simulink model "ofdm_txrx_supermimo_coded"  (Simulink 7.1)
settings: Solver=VariableStepDiscrete  SolverMode=SingleTasking  StartTime=0.0  StopTime=2*simOnly_simLength  RelTol=1e-3
PostLoadFcn:
  | ofdm_rx_supermimo_init
InitFcn:
  | ofdm_rx_supermimo_init
StartFcn:
  | ofdm_rx_supermimo_init
values from neighbouring files (symbols this model uses):
  Action0_Config = 0* 31*2^18 + 0*2^24 + PHY_AUTORESPONSE_REQ_GOODHDR + PHY_AUTORESPONSE_REQ_GOODPKT + PHY_AUTORESPONSE_REQ_FLAGA    [ofdm_tx_supermimo_init.m]
  Action1_Config = 0* 62*2^18 + PHY_AUTORESPONSE_REQ_GOODHDR + PHY_AUTORESPONSE_REQ_GOODPKT    [ofdm_tx_supermimo_init.m]
  Action2_Config = 0    [ofdm_tx_supermimo_init.m]
  Action3_Config = 0    [ofdm_tx_supermimo_init.m]
  Action4_Config = 0    [ofdm_tx_supermimo_init.m]
  Action5_Config = 0    [ofdm_tx_supermimo_init.m]
  ActionID_AFTransmit = 31    [ofdm_tx_supermimo_init.m]
  ActionID_Disabled = 0    [ofdm_tx_supermimo_init.m]
  ActionID_SetFlagA = 62    [ofdm_tx_supermimo_init.m]
  ActionID_SetFlagB = 61    [ofdm_tx_supermimo_init.m]
  BER_RAM_init_size = 2048    [ofdm_tx_supermimo_init.m]
  channel_h = [1 0; 1 0] * 0.3    [ofdm_tx_supermimo_init.m]
  CPLength = 16    [ofdm_tx_supermimo_init.m]
  FEC_Config = fec_coding_en*1 + fec_soft_dec*2 + fec_qpsk_scl*2^4 + fec_16qam_scl*2^8    (= 4195)    [ofdm_tx_supermimo_init.m]
  longCorr_coef_i = [-4*real(LTS) 0]    [ofdm_rx_supermimo_init.m]
  longCorr_coef_q = [4*imag(LTS) 0]    [ofdm_rx_supermimo_init.m]
  Match0_Config = 0    [ofdm_tx_supermimo_init.m]
  Match1_Config = 0    [ofdm_tx_supermimo_init.m]
  Match2_Config = 0    [ofdm_tx_supermimo_init.m]
  Match3_Config = 0    [ofdm_tx_supermimo_init.m]
  Match4_Config = 0    [ofdm_tx_supermimo_init.m]
  Match5_Config = 0    [ofdm_tx_supermimo_init.m]
  Match6_Config = 0    [ofdm_tx_supermimo_init.m]
  Match7_Config = 0    [ofdm_tx_supermimo_init.m]
  max_CP_length = 16    [ofdm_tx_supermimo_init.m]
  max_num_baseRateSymbols = 31    [ofdm_tx_supermimo_init.m]
  max_num_subcarriers = 64    [ofdm_tx_supermimo_init.m]
  max_num_trainingSymbols = 15    [ofdm_tx_supermimo_init.m]
  max_numBytes = 16384    [ofdm_tx_supermimo_init.m]
  max_OFDM_symbols = 1023    [ofdm_tx_supermimo_init.m]
  modConstellation_qam16 = .75*[1 3 -1 -3]./3    [ofdm_tx_supermimo_init.m]
  modConstellation_qam64 = 0.875*[3 1 5 7 -3 -1 -5 -7]./7    [ofdm_tx_supermimo_init.m]
  modConstellation_qpsk = [1 -1]./sqrt(2)    [ofdm_tx_supermimo_init.m]
  numBaseRateSymbols = numBaseRateSymbols *2    [ofdm_tx_supermimo_init.m]
  numSubcarriers = 64    [ofdm_tx_supermimo_init.m]
  numTrainingSymbols = 2    [ofdm_tx_supermimo_init.m]
  pilot_indicies = 7 + ( (64-21) * 2^8) + ( (64-7) * 2^16) + (21 * 2^24)    (= 356068103)    [ofdm_tx_supermimo_init.m]
  pilotValues = hex2dec('A57D') + (2^16 * hex2dec('5A82'))    [ofdm_tx_supermimo_init.m]
  pktByteNums = numHeaderBytes +  				byteIndex_numPayloadBytes(1)*2^8 +  				byteIndex_numPayloadBytes(2)*2^16 +  				byteIndex_simpleDynModMasks * 2^24    [ofdm_rx_supermimo_init.m]
  PktDet_Delay = 58+32 +      Symbol_Timing_Offset*2^7 +      0*2^16 +      (117* 2^24)    (= 1962935642)    [ofdm_rx_supermimo_init.m]
  pktTiming_count_payloadStart = pktTiming_count_lsStart + pktTiming_ls_num*pktTiming_ls_length    [ofdm_rx_supermimo_init.m]
  preamble = preamble_scale*[shortsyms_10 longsyms_2]    [ofdm_tx_supermimo_init.m]
  preamble_ROM = preamble_I_ROM + preamble_Q_ROM*2^16    [ofdm_tx_supermimo_init.m]
  RAM_init_size = max_numBytes    (= 16384)    [ofdm_tx_supermimo_init.m]
  reg_AF_Tx_Blanking = (320+80) + ( (320+80+79)*2^16)    (= 31392144)    [ofdm_rx_supermimo_init.m]
  reg_AF_Tx_Scaling = 2944    [ofdm_rx_supermimo_init.m]
  reg_Interrupt_PktBuf_Control = reg_InterruptControl +  	1 * 2^16 +  	0 * 2^24    (= 65536)    [ofdm_rx_supermimo_init.m]
  reg_PilotCalcParams = (4 * 2^16) + (minPilotChanMag)    (= 262184.96)    [ofdm_rx_supermimo_init.m]
  regRx_chanEst_minMag = ((0.5)*2^15) + ((0.5 * 2^15)*2^16)    (= 1073758208)    [ofdm_rx_supermimo_init.m]
  regRx_coarseCalcCorrection = round((1.3e-3 - 1.2982e-3)*2^32)    [ofdm_rx_supermimo_init.m]
  regRx_pilotCalcCorrection = round(1.00115 * 2^31)    [ofdm_rx_supermimo_init.m]
  regRx_preCFOoptions = 0 * 2^0 +  	1 * 2^1 +  	0    (= 2)    [ofdm_rx_supermimo_init.m]
  rx_controlBits = 1 * 2  		 + 1 * 4  		 + 0 * 8  		 + 1 * 16 * rx_SISO_Mode  		 + 1 * 32  		 + 1 * 64  		 + 1 * 128  		 + 1 * 256  		 + 1 * 512  		 + 1 * 1024  		 + 1 * 4096  		 + 0 * 16384  		 + 0 * 32768  		 + 1 * 65536  		 + 0 * 131072  		 + 0 * 262144  		 + 1 * 524288  		 + 1 * 1048576 * tx_Alamouti_Mode  		 + 0 * 2097152           + 1 * 33554432  		 + 0 * 4194304  		 + 0 * 8388608  		 + 0 * 16777216  		 + 1 *  ...(+49 chars)    [ofdm_rx_supermimo_init.m]
  Rx_PktDet_LongCorr_Params = LongCorrCounterLoad + 0*2^8 + 0*2^16 + 255*2^24    (= 4278190325)    [ofdm_rx_supermimo_init.m]
  Rx_PktDet_LongCorrThresholds = 8000    [ofdm_rx_supermimo_init.m]
  rx_postEq_scaling = round(rxScaling*2^11) * (1 + 2^16)    [ofdm_rx_supermimo_init.m]
  RxDataScrambling_Seq = [40 198 78 63 82 173 102 245 48 111 172 115 147 230 216 93 72 65 62 2 205 242 122 90 128 83 105 97 73 10 5 252]    [ofdm_tx_supermimo_init.m]
  RxReg_FixedPktLen = 0    [ofdm_rx_supermimo_init.m]
  rxReg_pktDetCorr = 2^00 * (floor(0.7*128)) +  	2^08 * (300) +  	0    [ofdm_rx_supermimo_init.m]
  shortSymbol_time = shortSymbol_time(1:16).'    [ofdm_tx_supermimo_init.m]
  simOnly_numSamples = length(preamble)+( (numSubcarriers+CPLength)*(numTrainingSymbols + numBaseRateSymbols + numFullRateSymbols/2) )    [ofdm_tx_supermimo_init.m]
  train = [train train]    [ofdm_tx_supermimo_init.m]
  tx_controlBits = (antB_preambleShift * 2^4) + (2^1 * tx_Alamouti_Mode) +  	(2^0 * tx_SISO_Mode) + 4*0 + 8*1 + 0*256 + 512*0 + 1024*0 +  	0*hex2dec('1000') + 0*hex2dec('2000') + 0*hex2dec('4000') +  	1*hex2dec('8000') + 1*hex2dec('10000') + hex2dec('20000') +  	0*hex2dec('100000')    [ofdm_tx_supermimo_init.m]
  tx_delays = (50*2^0) + (0*2^8) + (30*2^12)    (= 122930)    [ofdm_tx_supermimo_init.m]
  tx_scaling = (3072) + (12288 * 2^16)    (= 805309440)    [ofdm_tx_supermimo_init.m]
  TxDataScrambling_Seq = [40 198 78 63 82 173 102 245 48 111 172 115 147 230 216 93 72 65 62 2 205 242 122 90 128 83 105 97 73 10 5 252]    [ofdm_tx_supermimo_init.m]
  txReg_symbolCounts = (2^8 *numBaseRateSymbols ) + numTrainingSymbols    [ofdm_tx_supermimo_init.m]
  TxRx_FFTScaling = (3 * 2^0) +  	(2 * 2^2) +  	(1 * 2^4) +  	(1 * 2^6) +  	(1 * 2^8) +  	(0 * 2^10)    (= 347)    [ofdm_tx_supermimo_init.m]
undefined symbols (not defined in the file or in its folder): AntA, AntB, BaseRate, Down, gcbh, numBuffs, numBytes, Up, xlBlockLoadCallback, xlGetSimulinkPeriod, xlipgetpartsetting
libraries: xbsIndex_r4 x6000, simulink x4, dspsigops x4, commchan3 x2, dspsrcs4 x2, commrflib2 x2
(part 40 of 63)

== system ofdm_txrx_supermimo_coded/OFDM Tx MIMO/Training_Data/FlexibleMod/PktBuffer_CRC1/CRC32 Calc ==
  ByteIn : Inport  OutDataType=sfix(16)  OutScaling=2^0
  Vin : Inport  Orientation=left  Port=2  OutDataType=sfix(16)  OutScaling=2^0
  Rst : Inport  Orientation=left  Port=3  OutDataType=sfix(16)  OutScaling=2^0
  Capt : Inport  Port=4  OutDataType=sfix(16)  OutScaling=2^0
  24LSB : xbsIndex_r4/Slice  nbits=24  boolean_output=off  mode=Lower Bit Location + Width  bit1=0  base1=MSB of Input  bit0=0  base0=LSB of Input  dbl_ovrd=off  block_type=slice
      settings: infoedit="Extracts a given range of bits from each input sample and presents it at the output.  The output type is ordinarily unsigned with binary point at zero, but can be Boolean when the slice is one bit wide.<P><P>Hardware notes: In hardware this block costs nothing."  block_version=VER_STRING_GOES_HERE
  8MSB : xbsIndex_r4/Slice  nbits=8  boolean_output=off  mode=Upper Bit Location + Width  bit1=0  base1=MSB of Input  bit0=0  base0=LSB of Input  dbl_ovrd=off  block_type=slice
      settings: infoedit="Extracts a given range of bits from each input sample and presents it at the output.  The output type is ordinarily unsigned with binary point at zero, but can be Boolean when the slice is one bit wide.<P><P>Hardware notes: In hardware this block costs nothing."  block_version=VER_STRING_GOES_HERE
  Assert : xbsIndex_r4/Assert  Orientation=left  assert_type=off  type_source=Explicitly  arith_type="Signed  (2's comp)"  n_bits=16  bin_pt=14  assert_rate=on  rate_source=Explicitly  period=2  output_port=on  block_type=assert
      settings: infoedit=Asserts a user-defined sample rate and/or type on a signal.<P><P>Hardware notes: In hardware this block costs nothing.  block_version=VER_STRING_GOES_HERE
  CRC Remainders : xbsIndex_r4/ROM  depth=256  initVector=CRC_Table32  distributed_mem=Distributed memory  rst=off  init_reg=0  en=off  latency=0  arith_type=Unsigned  n_bits=32  bin_pt=0  dbl_ovrd=off  optimize=Area  use_rpm=off  block_type=sprom
      settings: xl_area=[0, 0, 0, 0, 0, 0, 0]  block_version=VER_STRING_GOES_HERE
  Concat : xbsIndex_r4/Concat  num_inputs=2  dbl_ovrd=off  block_type=concat
      settings: block_version=VER_STRING_GOES_HERE
  Constant : xbsIndex_r4/Constant  arith_type=Unsigned  const=0  n_bits=8  bin_pt=0  explicit_period=off  period=1  equ=P=C  opselect=C  inp2=PCIN>>17  opr=+  inp1=P  carry=CIN  dbl_ovrd=off  block_type=constant
      settings: block_version=VER_STRING_GOES_HERE
  Delay : xbsIndex_r4/Delay  en=off  latency=1  dbl_ovrd=off  reg_retiming=off  block_type=delay
      settings: infoedit=Hardware notes: A delay line is a chain, each link of which is an SRL16 followed by a flip-flop. If register retiming is enabled, the delay line is a chain of flip-flops.  block_version=8.2.01
  Logical1 : xbsIndex_r4/Logical  logical_function=XOR  inputs=2  en=off  latency=0  precision=Full  arith_type=Unsigned  n_bits=8  bin_pt=2  align_bp=on  dbl_ovrd=off  block_type=logical
      settings: xl_area=[0, 0, 0, 0, 0, 0, 0]  block_version=VER_STRING_GOES_HERE
  Logical2 : xbsIndex_r4/Logical  Orientation=left  logical_function=XOR  inputs=2  en=off  latency=0  precision=Full  arith_type=Unsigned  n_bits=8  bin_pt=2  align_bp=on  dbl_ovrd=off  block_type=logical
      settings: xl_area=[0, 0, 0, 0, 0, 0, 0]  block_version=VER_STRING_GOES_HERE
  Logical4 : xbsIndex_r4/Logical  logical_function=AND  inputs=2  en=off  latency=0  precision=Full  arith_type=Unsigned  n_bits=8  bin_pt=2  align_bp=on  dbl_ovrd=off  block_type=logical
      settings: xl_area=[1 0 0 1 0 0 0]  block_version=VER_STRING_GOES_HERE
  crc_accum : xbsIndex_r4/Register  Orientation=left  init=hex2dec('FFFFFFFF')  rst=on  en=on  dbl_ovrd=off  block_type=register
      settings: xl_area=[0, 0, 0, 0, 0, 0, 0]  block_version=VER_STRING_GOES_HERE
  crc_capt : xbsIndex_r4/Register  init=0  rst=on  en=on  dbl_ovrd=off  block_type=register
      settings: xl_area=[0, 0, 0, 0, 0, 0, 0]  block_version=VER_STRING_GOES_HERE
  32-bit CRC Capt : Outport  OutDataType=sfix(16)  OutScaling=2^0
  wires:
  8MSB/1 -> Logical1/1
  crc_accum/1 -> Assert/1
  Logical1/1 -> CRC Remainders/1
  Logical2/1 -> crc_accum/1, crc_capt/1
  24LSB/1 -> Concat/1
  Constant/1 -> Concat/2
  Concat/1 -> Logical2/1
  CRC Remainders/1 -> Logical2/2
  Rst/1 -> crc_accum/2, crc_capt/2
  ByteIn/1 -> Logical1/2
  Vin/1 -> crc_accum/3, Logical4/2
  Assert/1 -> 24LSB/1, 8MSB/1
  Logical4/1 -> Delay/1
  Capt/1 -> Logical4/1
  crc_capt/1 -> 32-bit CRC Capt/1
  Delay/1 -> crc_capt/3

== system ofdm_txrx_supermimo_coded/OFDM Tx MIMO/Training_Data/FlexibleMod/PktBuffer_CRC1/Header Decoding1 ==
  TxByteAddr : Inport  OutDataType=sfix(16)  OutScaling=2^0
  TxByte : Inport  Port=2  OutDataType=sfix(16)  OutScaling=2^0
  TxByteAddrVin : Inport  Port=3  OutDataType=sfix(16)  OutScaling=2^0
  Rst : Inport  Port=4  OutDataType=sfix(16)  OutScaling=2^0
  4LSB : xbsIndex_r4/Slice  nbits=4  boolean_output=off  mode=Lower Bit Location + Width  bit1=0  base1=MSB of Input  bit0=0  base0=LSB of Input  dbl_ovrd=off  block_type=slice
      settings: infoedit="Extracts a given range of bits from each input sample and presents it at the output.  The output type is ordinarily unsigned with binary point at zero, but can be Boolean when the slice is one bit wide.<P><P>Hardware notes: In hardware this block costs nothing."  block_version=VER_STRING_GOES_HERE
  4MSB : xbsIndex_r4/Slice  nbits=4  boolean_output=off  mode=Upper Bit Location + Width  bit1=0  base1=MSB of Input  bit0=0  base0=LSB of Input  dbl_ovrd=off  block_type=slice
      settings: infoedit="Extracts a given range of bits from each input sample and presents it at the output.  The output type is ordinarily unsigned with binary point at zero, but can be Boolean when the slice is one bit wide.<P><P>Hardware notes: In hardware this block costs nothing."  block_version=VER_STRING_GOES_HERE
  8LSB : xbsIndex_r4/Slice  nbits=8  boolean_output=off  mode=Lower Bit Location + Width  bit1=0  base1=MSB of Input  bit0=0  base0=LSB of Input  dbl_ovrd=off  block_type=slice
      settings: infoedit="Extracts a given range of bits from each input sample and presents it at the output.  The output type is ordinarily unsigned with binary point at zero, but can be Boolean when the slice is one bit wide.<P><P>Hardware notes: In hardware this block costs nothing."  block_version=VER_STRING_GOES_HERE
  8LSB+8 : xbsIndex_r4/Slice  nbits=8  boolean_output=off  mode=Lower Bit Location + Width  bit1=0  base1=MSB of Input  bit0=8  base0=LSB of Input  dbl_ovrd=off  block_type=slice
      settings: infoedit="Extracts a given range of bits from each input sample and presents it at the output.  The output type is ordinarily unsigned with binary point at zero, but can be Boolean when the slice is one bit wide.<P><P>Hardware notes: In hardware this block costs nothing."  block_version=VER_STRING_GOES_HERE
  8MSB : xbsIndex_r4/Slice  nbits=8  boolean_output=off  mode=Upper Bit Location + Width  bit1=0  base1=MSB of Input  bit0=0  base0=LSB of Input  dbl_ovrd=off  block_type=slice
      settings: infoedit="Extracts a given range of bits from each input sample and presents it at the output.  The output type is ordinarily unsigned with binary point at zero, but can be Boolean when the slice is one bit wide.<P><P>Hardware notes: In hardware this block costs nothing."  block_version=VER_STRING_GOES_HERE
  Assert1 : xbsIndex_r4/Assert  assert_type=off  type_source=Explicitly  arith_type="Signed  (2's comp)"  n_bits=16  bin_pt=14  assert_rate=on  rate_source=Explicitly  period=2  output_port=on  block_type=assert
      settings: infoedit=Asserts a user-defined sample rate and/or type on a signal.<P><P>Hardware notes: In hardware this block costs nothing.  block_version=VER_STRING_GOES_HERE
  Assert2 : xbsIndex_r4/Assert  assert_type=off  type_source=Explicitly  arith_type="Signed  (2's comp)"  n_bits=16  bin_pt=14  assert_rate=on  rate_source=Explicitly  period=2  output_port=on  block_type=assert
      settings: infoedit=Asserts a user-defined sample rate and/or type on a signal.<P><P>Hardware notes: In hardware this block costs nothing.  block_version=VER_STRING_GOES_HERE
  Logical1 : xbsIndex_r4/Logical  logical_function=AND  inputs=2  en=off  latency=0  precision=Full  arith_type=Unsigned  n_bits=8  bin_pt=2  align_bp=on  dbl_ovrd=off  block_type=logical
      settings: xl_area=[1 0 0 1 0 0 0]  block_version=VER_STRING_GOES_HERE
  Logical2 : xbsIndex_r4/Logical  logical_function=AND  inputs=2  en=off  latency=0  precision=Full  arith_type=Unsigned  n_bits=8  bin_pt=2  align_bp=on  dbl_ovrd=off  block_type=logical
      settings: xl_area=[1 0 0 1 0 0 0]  block_version=VER_STRING_GOES_HERE
  Logical4 : xbsIndex_r4/Logical  logical_function=AND  inputs=2  en=off  latency=0  precision=Full  arith_type=Unsigned  n_bits=8  bin_pt=2  align_bp=on  dbl_ovrd=off  block_type=logical
      settings: xl_area=[1 0 0 1 0 0 0]  block_version=VER_STRING_GOES_HERE
  Register1 : xbsIndex_r4/Register  init=34  rst=on  en=on  dbl_ovrd=off  block_type=register
      settings: xl_area=[5 10 0 0 0 0 0]  block_version=VER_STRING_GOES_HERE
  Register2 : xbsIndex_r4/Register  init=0  rst=on  en=off  dbl_ovrd=off  block_type=register
      settings: xl_area=[5 10 0 0 0 0 0]  block_version=VER_STRING_GOES_HERE
  Relational1 : xbsIndex_r4/Relational  mode=a=b  en=off  latency=0  dbl_ovrd=off  block_type=relational
      settings: xl_area=[6 0 0 11 0 0 0]  block_version=VER_STRING_GOES_HERE
  Relational2 : xbsIndex_r4/Relational  mode=a=b  en=off  latency=0  dbl_ovrd=off  block_type=relational
      settings: xl_area=[6 0 0 11 0 0 0]  block_version=VER_STRING_GOES_HERE
  Relational3 : xbsIndex_r4/Relational  mode=a=b  en=off  latency=0  dbl_ovrd=off  block_type=relational
      settings: xl_area=[6 0 0 11 0 0 0]  block_version=VER_STRING_GOES_HERE
  numBytesUpdate : Subsystem
  numBytes : Outport  OutDataType=sfix(16)  OutScaling=2^0
  modMask : Outport  Port=2  OutDataType=sfix(16)  OutScaling=2^0
  wires:
  Relational3/1 -> Logical2/2
  8LSB+8/1 -> Relational3/2
  Relational2/1 -> Logical4/2
  8LSB/1 -> Relational2/2
  Rst/1 -> Register1/2, numBytesUpdate/1(rst), Register2/2
  Logical4/1 -> numBytesUpdate/3(lsb_EN)
  Logical2/1 -> numBytesUpdate/4(msb_EN)
  numBytesUpdate/1(numBytes) -> Assert1/1
  Assert1/1 -> numBytes/1
  [regRx_headerByteNums] -> 8LSB+8/1, 8LSB/1, 8MSB/1
  TxByteAddrVin/1 -> Logical4/1, Logical1/1, Logical2/1
  TxByte/1 -> numBytesUpdate/2(d), Register1/1
  Register2/1 -> Relational2/1, Relational3/1, Relational1/1
  Relational1/1 -> Logical1/2
  Logical1/1 -> Register1/3
  8MSB/1 -> Relational1/2
  Register1/1 -> Assert2/1
  Assert2/1 -> modMask/1, 4LSB/1, 4MSB/1
  4LSB/1 -> numBytesUpdate/6(modMask_AntB)
  4MSB/1 -> numBytesUpdate/5(modMask_AntA)
  TxByteAddr/1 -> Register2/1

== system ofdm_txrx_supermimo_coded/OFDM Tx MIMO/Training_Data/FlexibleMod/PktBuffer_CRC1/Packet Buffer ==
  note: For real-time BER testing, the Rx block uses this logic
      | to access the packet buffer. In hardware, Tx/Rx is half-duplex,
      | so sharing the logic is no problem. In simulation, it's full-duplex
      | (loopback, really), so some muxing is required.
  note: Use a long (12-bit) LFSR for more random payloads
      | Delay the Mux select by 1 cycle to line up with the latency
      | of the actual packet buffer (don't want to randomize the last
      | byte of the header!)
      | Polynomial set to dec2hex(2^11+2^8+2^6+2^0),
      | the optimal for a 12-bit LFSR per xapp210.
  note: Increment the LFSR on each new byte address. This
      | logic will "miss" byte0, but since we don't record
      | headers for random payloads, it doesn't matter.
  note: Disable random payload generation for
      | automatically transmitted packets
      | (either via autoTwoTx or via
      | autoResponders)
  TxByteAddr : Inport  OutDataType=sfix(16)  OutScaling=2^0
  1LSB : xbsIndex_r4/Slice  nbits=1  boolean_output=on  mode=Lower Bit Location + Width  bit1=0  base1=MSB of Input  bit0=0  base0=LSB of Input  dbl_ovrd=off  block_type=slice
      settings: infoedit="Extracts a given range of bits from each input sample and presents it at the output.  The output type is ordinarily unsigned with binary point at zero, but can be Boolean when the slice is one bit wide.<P><P>Hardware notes: In hardware this block costs nothing."  block_version=8.2.02
  8LSB : xbsIndex_r4/Slice  nbits=8  boolean_output=off  mode=Lower Bit Location + Width  bit1=0  base1=MSB of Input  bit0=0  base0=LSB of Input  dbl_ovrd=off  block_type=slice
      settings: infoedit="Extracts a given range of bits from each input sample and presents it at the output.  The output type is ordinarily unsigned with binary point at zero, but can be Boolean when the slice is one bit wide.<P><P>Hardware notes: In hardware this block costs nothing."  block_version=8.2.02
  AutoTx Header Translation : Subsystem
  Convert{,1..3} (4 alike) : xbsIndex_r4/Convert  arith_type=Boolean  n_bits=16  bin_pt=14  quantization=Truncate  overflow=Wrap  latency=0  dbl_ovrd=off  pipeline=off  block_type=convert
      settings: block_version=10.1.3
  Delay{,1,3..6} (6 alike) : xbsIndex_r4/Delay  en=off  latency=1  dbl_ovrd=off  reg_retiming=off  block_type=delay
  Down Sample : xbsIndex_r4/Down Sample  sample_ratio=2  sample_phase="Last Value of Frame  (most efficient)"  en=off  latency=1  dbl_ovrd=off  block_type=dsamp
      settings: block_version=8.2.02
  Down Sample1 : xbsIndex_r4/Down Sample  sample_ratio=2  sample_phase="Last Value of Frame  (most efficient)"  en=off  latency=1  dbl_ovrd=off  block_type=dsamp
      settings: block_version=8.2.02
  Inverter1 : xbsIndex_r4/Inverter  en=off  latency=0  dbl_ovrd=off  block_type=inv
  Inverter2 : xbsIndex_r4/Inverter  en=off  latency=0  dbl_ovrd=off  block_type=inv
  Inverter3 : xbsIndex_r4/Inverter  en=off  latency=0  dbl_ovrd=off  block_type=inv
  LFSR : xbsIndex_r4/LFSR  lfsr_type=Fibonacci  gate_type=XOR  n_bits=12  polynomial=dec2hex(2^11+2^8+2^6+2^0)  rst_value='1'  rst=off  en=on  reloadable_seed=off  input_type=off  output_type=on  arith_type=Unsigned  bin_pt=0  dbl_ovrd=off  explicit_period=off  period=1  block_type=lfsr
      settings: block_version=10.1.3
  Logical1 : xbsIndex_r4/Logical  logical_function=AND  inputs=4  en=off  latency=0  precision=Full  arith_type=Unsigned  n_bits=8  bin_pt=2  align_bp=on  dbl_ovrd=off  block_type=logical
      settings: xl_area=[0, 0, 0, 0, 0, 0, 0]  block_version=VER_STRING_GOES_HERE
  Logical2 : xbsIndex_r4/Logical  logical_function=XOR  inputs=2  en=off  latency=0  precision=Full  arith_type=Unsigned  n_bits=8  bin_pt=2  align_bp=on  dbl_ovrd=off  block_type=logical
      settings: xl_area=[0, 0, 0, 0, 0, 0, 0]  block_version=VER_STRING_GOES_HERE
  Logical3 : xbsIndex_r4/Logical  logical_function=AND  inputs=2  en=off  latency=0  precision=Full  arith_type=Unsigned  n_bits=8  bin_pt=2  align_bp=on  dbl_ovrd=off  block_type=logical
      settings: xl_area=[0, 0, 0, 0, 0, 0, 0]  block_version=VER_STRING_GOES_HERE
  Mux1 : xbsIndex_r4/Mux  inputs=2  en=off  latency=0  precision=Full  arith_type=Unsigned  n_bits=16  bin_pt=14  quantization=Truncate  overflow=Wrap  dbl_ovrd=off  block_type=mux
      settings: block_version=8.2.02
  Mux2 : xbsIndex_r4/Mux  inputs=2  en=off  latency=0  precision=Full  arith_type=Unsigned  n_bits=16  bin_pt=14  quantization=Truncate  overflow=Wrap  dbl_ovrd=off  block_type=mux
      settings: block_version=8.2.02
  Pkt Buf IF : Subsystem
  Simulation Multiplexer : xbsIndex_r4/Simulation Multiplexer  sim_sel=1  hw_sel=2  block_type=simmux
      settings: infoedit="Distinguish input subsystems as \"simulation only\" and \"generation only\".  The input specified For Simulation will be used during Simulink simulation.  The input specified For Generation will be used during code generation.  This block will typically be used on the output of a HW Co-Simulation, Black Box, or ModelSim subsystem.<P><P>Hardware Notes: This block costs nothing."  block_version=10.1.3
  Tx Pkt Buf : Scope  NumInputPorts=8
      settings: TimeRange=8000  YMin=0~0~0~0~0~0~0~0  YMax=80~80~200~300~1~1~80~1  SaveName=ScopeData16
  Up Sample7 : xbsIndex_r4/Up Sample  sample_ratio=2  copy_samples=on  dbl_ovrd=off  block_type=usamp
      settings: infoedit="Up samples input data.  Inserted values  can be zeros or copies of the most recent input sample.<P><P>Hardware notes: No hardware is needed if inserted values are copies of the input sample; otherwise, a mux and single bit flip-flop are used."  block_version=8.2.02
  done{1..7} (7 alike) : xbsIndex_r4/Gateway Out  hdl_port=off  timing_constraint=None  locs_specified=off  LOCs={}  block_type=gatewayout
      settings: infoedit="Gateway out block.  Converts Xilinx fixed point inputs into ouputs of type Simulink integer, double, or fixed point.<P><P>Hardware notes:  In hardware these blocks become top level output ports or are discarded, depending on how they are configured."  xl_area=[0 0 0 0 1 0 0]  block_version=VER_STRING_GOES_HERE
  ByteOut : Outport  OutDataType=sfix(16)  OutScaling=2^0
  tag scope: Tx_RandomPayloadByte=global, Tx_RandomPayloadByte_addr=global, Tx_RandomPayloadByte_valid=global
  wires:
  [regRx_FlexBERMode] -> Mux1/1
  [FlexBER_BRAM_Rx_ByteWrAddr] -> Mux1/3
  Mux1/1 -> Simulation Multiplexer/2
  Up Sample7/1 -> Mux1/2, Simulation Multiplexer/1
  AutoTx Header Translation/1(" ByteAddr") -> Up Sample7/1, done5/1
  Simulation Multiplexer/1 -> Pkt Buf IF/1(ByteAddr)
  Pkt Buf IF/1(ByteOut) -> Mux2/2, done3/1
  TxByteAddr/1 -> AutoTx Header Translation/1(ByteAddr), done4/1, 1LSB/1, Delay5/1
  AutoTx Header Translation/2(Header) -> Inverter2/1
  [regTx_RandomPayload] -> Convert1/1
  Logical1/1 -> Delay/1, Logical3/1
  Mux2/1 -> ByteOut/1
  Inverter2/1 -> Convert/1
  Convert/1 -> Logical1/1
  Convert1/1 -> Logical1/2
  LFSR/1 -> 8LSB/1
  8LSB/1 -> [Tx_RandomPayloadByte], Mux2/3, done6/1
  Delay/1 -> Mux2/1
  Delay1/1 -> [Tx_RandomPayloadByte_valid], done2/1
  1LSB/1 -> Logical2/1, Delay3/1
  Delay3/1 -> Logical2/2
  Logical3/1 -> Delay1/1, LFSR/1, done1/1
  Logical2/1 -> Delay4/1
  done4/1 -> Tx Pkt Buf/1
  done5/1 -> Tx Pkt Buf/2
  done3/1 -> Tx Pkt Buf/3
  done6/1 -> Tx Pkt Buf/4
  done1/1 -> Tx Pkt Buf/5
  Delay4/1 -> Logical3/2
  Delay5/1 -> Delay6/1
  Delay6/1 -> [Tx_RandomPayloadByte_addr], done7/1
  done2/1 -> Tx Pkt Buf/6
  done7/1 -> Tx Pkt Buf/7
  Inverter1/1 -> Convert2/1
  Convert2/1 -> Logical1/3
  [RxAutoReply_AutoTx] -> Down Sample/1
  [RxAutoReply_ExtTxEn] -> Down Sample1/1
  Inverter3/1 -> Convert3/1
  Convert3/1 -> Logical1/4
  Down Sample/1 -> Inverter1/1
  Down Sample1/1 -> Inverter3/1

== system ofdm_txrx_supermimo_coded/OFDM Tx MIMO/Training_Data/FlexibleMod/PktBuffer_CRC1/Scrambling ==
  Din : Inport  OutDataType=sfix(16)  OutScaling=2^0
  Addr : Inport  Port=2  OutDataType=sfix(16)  OutScaling=2^0
  5LSB : xbsIndex_r4/Slice  nbits=ceil(log2(length(TxDataScrambling_Seq)))  boolean_output=off  mode=Lower Bit Location + Width  bit1=0  base1=MSB of Input  bit0=0  base0=LSB of Input  dbl_ovrd=off  block_type=slice
      settings: infoedit="Extracts a given range of bits from each input sample and presents it at the output.  The output type is ordinarily unsigned with binary point at zero, but can be Boolean when the slice is one bit wide.<P><P>Hardware notes: In hardware this block costs nothing."  block_version=8.2.02
  Logical : xbsIndex_r4/Logical  logical_function=XOR  inputs=2  en=off  latency=0  precision=Full  arith_type=Unsigned  n_bits=16  bin_pt=0  align_bp=on  dbl_ovrd=off  block_type=logical
      settings: block_version=8.2.02
  ROM : xbsIndex_r4/ROM  depth=length(TxDataScrambling_Seq)  initVector=TxDataScrambling_Seq  distributed_mem=Distributed memory  rst=off  init_reg=0  en=off  latency=1  arith_type=Unsigned  n_bits=8  bin_pt=0  dbl_ovrd=off  optimize=Area  use_rpm=on  block_type=sprom
      settings: block_version=8.2.02
  Dout : Outport  OutDataType=sfix(16)  OutScaling=2^0
  wires:
  ROM/1 -> Logical/2
  5LSB/1 -> ROM/1
  Logical/1 -> Dout/1
  Din/1 -> Logical/1
  Addr/1 -> 5LSB/1

== system ofdm_txrx_supermimo_coded/OFDM Tx MIMO/Training_Data/FlexibleMod/QAM/QPSK Map ==
  Bit : Inport  OutDataType=sfix(16)  OutScaling=2^0
  Constant6 : xbsIndex_r4/Constant  arith_type=Signed (2's comp)  const=modConstellation_qpsk(1)  n_bits=modConstellation_prec  bin_pt=modConstellation_bp  explicit_period=off  period=1  equ=P=C  opselect=C  inp2=PCIN>>17  opr=+  inp1=P  carry=CIN  dbl_ovrd=off  block_type=constant
      settings: block_version=VER_STRING_GOES_HERE
  Constant7 : xbsIndex_r4/Constant  arith_type=Signed (2's comp)  const=modConstellation_qpsk(2)  n_bits=modConstellation_prec  bin_pt=modConstellation_bp  explicit_period=off  period=1  equ=P=C  opselect=C  inp2=PCIN>>17  opr=+  inp1=P  carry=CIN  dbl_ovrd=off  block_type=constant
      settings: block_version=VER_STRING_GOES_HERE
  Mux2 : xbsIndex_r4/Mux  inputs=2  en=off  latency=1  precision=Full  arith_type="Signed  (2's comp)"  n_bits=8  bin_pt=2  quantization=Truncate  overflow=Wrap  dbl_ovrd=off  block_type=mux
      settings: xl_area=[4 8 0 8 0 0 0]  block_version=VER_STRING_GOES_HERE
  Sym : Outport  OutDataType=sfix(16)  OutScaling=2^0
  wires:
  Mux2/1 -> Sym/1
  Bit/1 -> Mux2/1
  Constant7/1 -> Mux2/3
  Constant6/1 -> Mux2/2

== system ofdm_txrx_supermimo_coded/OFDM Tx MIMO/Training_Data/FlexibleMod/QAM/QPSK Map1 ==
  Bit : Inport  OutDataType=sfix(16)  OutScaling=2^0
  Constant6 : xbsIndex_r4/Constant  arith_type=Signed (2's comp)  const=modConstellation_qpsk(1)  n_bits=modConstellation_prec  bin_pt=modConstellation_bp  explicit_period=off  period=1  equ=P=C  opselect=C  inp2=PCIN>>17  opr=+  inp1=P  carry=CIN  dbl_ovrd=off  block_type=constant
      settings: block_version=VER_STRING_GOES_HERE
  Constant7 : xbsIndex_r4/Constant  arith_type=Signed (2's comp)  const=modConstellation_qpsk(2)  n_bits=modConstellation_prec  bin_pt=modConstellation_bp  explicit_period=off  period=1  equ=P=C  opselect=C  inp2=PCIN>>17  opr=+  inp1=P  carry=CIN  dbl_ovrd=off  block_type=constant
      settings: block_version=VER_STRING_GOES_HERE
  Mux2 : xbsIndex_r4/Mux  inputs=2  en=off  latency=1  precision=Full  arith_type="Signed  (2's comp)"  n_bits=8  bin_pt=2  quantization=Truncate  overflow=Wrap  dbl_ovrd=off  block_type=mux
      settings: xl_area=[4 8 0 8 0 0 0]  block_version=VER_STRING_GOES_HERE
  Sym : Outport  OutDataType=sfix(16)  OutScaling=2^0
  wires:
  Constant6/1 -> Mux2/2
  Constant7/1 -> Mux2/3
  Bit/1 -> Mux2/1
  Mux2/1 -> Sym/1

== system ofdm_txrx_supermimo_coded/OFDM Tx MIMO/Training_Data/FlexibleMod/control/Concat1 ==
  in0 : Inport  OutDataType=sfix(16)  OutScaling=2^0
  in1 : Inport  Port=2  OutDataType=sfix(16)  OutScaling=2^0
  Concat1 : xbsIndex_r4/Concat  num_inputs=2  dbl_ovrd=off  block_type=concat
      settings: block_version=VER_STRING_GOES_HERE
  out0 : Outport  OutDataType=sfix(16)  OutScaling=2^0
  wires:
  in0/1 -> Concat1/1
  in1/1 -> Concat1/2
  Concat1/1 -> out0/1

== system ofdm_txrx_supermimo_coded/OFDM Tx MIMO/Training_Data/FlexibleMod/control/Tx Modulation ==
  note: In Alamouti mode, we need two IFFT frames of data,
      | followed by two empty frames. The oscillator here does this
      | by forcing mod=0 for empty frames. The oscillator is reset
      | with each new packet (by posedge(baseRate)).
      | In SISO/Multiplexing, all of this is unnecessary.
  BaseRate : Inport  OutDataType=sfix(16)  OutScaling=2^0
  Ant Sel : Inport  Port=2  OutDataType=sfix(16)  OutScaling=2^0
  IFFT Index : Inport  Port=3  OutDataType=sfix(16)  OutScaling=2^0
  FullRateMasks : Inport  Port=4  OutDataType=sfix(16)  OutScaling=2^0
  4LSB : xbsIndex_r4/Slice  nbits=4  boolean_output=off  mode=Lower Bit Location + Width  bit1=0  base1=MSB of Input  bit0=0  base0=LSB of Input  dbl_ovrd=off  block_type=slice
      settings: infoedit="Extracts a given range of bits from each input sample and presents it at the output.  The output type is ordinarily unsigned with binary point at zero, but can be Boolean when the slice is one bit wide.<P><P>Hardware notes: In hardware this block costs nothing."  block_version=VER_STRING_GOES_HERE
  4MSB : xbsIndex_r4/Slice  nbits=4  boolean_output=off  mode=Upper Bit Location + Width  bit1=0  base1=MSB of Input  bit0=0  base0=LSB of Input  dbl_ovrd=off  block_type=slice
      settings: infoedit="Extracts a given range of bits from each input sample and presents it at the output.  The output type is ordinarily unsigned with binary point at zero, but can be Boolean when the slice is one bit wide.<P><P>Hardware notes: In hardware this block costs nothing."  block_version=VER_STRING_GOES_HERE
  AntB Mod Logic : Subsystem
  Concat1 : xbsIndex_r4/Concat  num_inputs=4  dbl_ovrd=off  block_type=concat
      settings: block_version=VER_STRING_GOES_HERE
  Concat2 : xbsIndex_r4/Concat  num_inputs=4  dbl_ovrd=off  block_type=concat
      settings: block_version=VER_STRING_GOES_HERE
  Concat3 : xbsIndex_r4/Concat  num_inputs=3  dbl_ovrd=off  block_type=concat
      settings: block_version=VER_STRING_GOES_HERE
  Inverter1 : xbsIndex_r4/Inverter  en=off  latency=0  dbl_ovrd=off  block_type=inv
      settings: block_version=VER_STRING_GOES_HERE
  Logical : xbsIndex_r4/Logical  logical_function=AND  inputs=2  en=off  latency=0  precision=Full  arith_type=Unsigned  n_bits=16  bin_pt=0  align_bp=on  dbl_ovrd=off  block_type=logical
      settings: block_version=VER_STRING_GOES_HERE
  Logical1 : xbsIndex_r4/Logical  logical_function=OR  inputs=2  en=off  latency=0  precision=Full  arith_type=Unsigned  n_bits=8  bin_pt=2  align_bp=on  dbl_ovrd=off  block_type=logical
      settings: xl_area=[1 0 0 1 0 0 0]  block_version=VER_STRING_GOES_HERE
  Logical2 : xbsIndex_r4/Logical  logical_function=AND  inputs=2  en=off  latency=0  precision=Full  arith_type=Unsigned  n_bits=8  bin_pt=2  align_bp=on  dbl_ovrd=off  block_type=logical
      settings: xl_area=[1 0 0 1 0 0 0]  block_version=VER_STRING_GOES_HERE
  Logical3 : xbsIndex_r4/Logical  logical_function=AND  inputs=2  en=off  latency=0  precision=Full  arith_type=Unsigned  n_bits=8  bin_pt=2  align_bp=on  dbl_ovrd=off  block_type=logical
      settings: xl_area=[1 0 0 1 0 0 0]  block_version=VER_STRING_GOES_HERE
  Logical4 : xbsIndex_r4/Logical  logical_function=OR  inputs=3  en=off  latency=0  precision=Full  arith_type=Unsigned  n_bits=16  bin_pt=0  align_bp=on  dbl_ovrd=off  block_type=logical
      settings: block_version=VER_STRING_GOES_HERE
  Mux1 : xbsIndex_r4/Mux  inputs=2  en=off  latency=0  precision=Full  arith_type=Unsigned  n_bits=16  bin_pt=14  quantization=Truncate  overflow=Wrap  dbl_ovrd=off  block_type=mux
      settings: block_version=8.2.02
  Oscillator : Subsystem
  Shared Memory for Modulation Masks : Subsystem
  negedge : Subsystem
  posedge : Subsystem
  mod : Outport  OutDataType=sfix(16)  OutScaling=2^0
  wires:
  BaseRate/1 -> Concat2/1, Concat2/2, Concat2/3, Concat2/4, Concat3/1, posedge/1(d), AntB Mod Logic/1(BaseRate)
  IFFT Index/1 -> Concat3/3
  [TxReg_SISO_Mode] -> Logical2/2
  Concat3/1 -> Shared Memory for Modulation Masks/1(Addr)
  Logical/1 -> mod/1
  FullRateMasks/1 -> 4LSB/1, 4MSB/1
  4LSB/1 -> Mux1/2
  4MSB/1 -> Mux1/3
  Mux1/1 -> Logical4/2
  Logical4/1 -> Logical/1
  [regRx_simpleDynRxModulationEn] -> Inverter1/1
  Inverter1/1 -> Concat1/1, Concat1/2, Concat1/3, Concat1/4
  Concat2/1 -> Logical4/3
  Ant Sel/1 -> Mux1/1, Logical2/1, negedge/1(d), AntB Mod Logic/2(AntSel)
  Concat1/1 -> Logical4/1
  Shared Memory for Modulation Masks/1(ModMask) -> Logical/2
  Logical2/1 -> Logical1/1
  Logical1/1 -> Shared Memory for Modulation Masks/2(Rst)
  negedge/1(q) -> Oscillator/2(en)
  Logical3/1 -> Logical1/2
  [TxReg_Alamouti_Mode] -> Logical3/1
  Oscillator/1(Q) -> Logical3/2
  AntB Mod Logic/1(AntB Mod En) -> Concat3/2
  posedge/1(q) -> Oscillator/1(rst)

== system ofdm_txrx_supermimo_coded/OFDM Tx MIMO/Training_Data/FlexibleMod/control/ending ==
  signal : Inport  OutDataType=sfix(16)  OutScaling=2^0
  Delay2 : xbsIndex_r4/Delay  en=off  latency=1  dbl_ovrd=off  reg_retiming=off  block_type=delay
      settings: infoedit=Hardware notes: A delay line is a chain, each link of which is an SRL16 followed by a flip-flop. If register retiming is enabled, the delay line is a chain of flip-flops.  xl_area=[1 1 0 0 0 0 0]  block_version=VER_STRING_GOES_HERE
  Inverter3 : xbsIndex_r4/Inverter  en=off  latency=0  dbl_ovrd=off  block_type=inv
      settings: xl_area=[0 0 0 0 0 0 0]  block_version=VER_STRING_GOES_HERE
  Logical2 : xbsIndex_r4/Logical  logical_function=AND  inputs=2  en=off  latency=0  precision=Full  arith_type=Unsigned  n_bits=8  bin_pt=2  align_bp=on  dbl_ovrd=off  block_type=logical
      settings: xl_area=[1 0 0 1 0 0 0]  block_version=VER_STRING_GOES_HERE
  pulse : Outport  OutDataType=sfix(16)  OutScaling=2^0
  wires:
  Delay2/1 -> Logical2/2
  Inverter3/1 -> Delay2/1
  signal/1 -> Logical2/1, Inverter3/1
  Logical2/1 -> pulse/1

== system ofdm_txrx_supermimo_coded/OFDM Tx MIMO/Training_Data/FlexibleMod/posedge1/Delay3 ==
  in0 : Inport  OutDataType=sfix(16)  OutScaling=2^0
  Delay3 : xbsIndex_r4/Delay  en=off  latency=1  dbl_ovrd=off  reg_retiming=off  block_type=delay
      settings: infoedit=Hardware notes: A delay line is a chain, each link of which is an SRL16 followed by a flip-flop. If register retiming is enabled, the delay line is a chain of flip-flops.  xl_area=[1 1 0 0 0 0 0]  block_version=VER_STRING_GOES_HERE
  out0 : Outport  OutDataType=sfix(16)  OutScaling=2^0
  wires:
  in0/1 -> Delay3/1
  Delay3/1 -> out0/1

== system ofdm_txrx_supermimo_coded/OFDM Tx MIMO/Training_Data/FlexibleMod/posedge1/Inverter2 ==
  in0 : Inport  OutDataType=sfix(16)  OutScaling=2^0
  Inverter2 : xbsIndex_r4/Inverter  en=off  latency=0  dbl_ovrd=off  block_type=inv
      settings: xl_area=[0 0 0 0 0 0 0]  block_version=VER_STRING_GOES_HERE
  out0 : Outport  OutDataType=sfix(16)  OutScaling=2^0
  wires:
  in0/1 -> Inverter2/1
  Inverter2/1 -> out0/1

== system ofdm_txrx_supermimo_coded/OFDM Tx MIMO/Training_Data/FlexibleMod/posedge1/Logical3 ==
  in0 : Inport  OutDataType=sfix(16)  OutScaling=2^0
  in1 : Inport  Port=2  OutDataType=sfix(16)  OutScaling=2^0
  Logical3 : xbsIndex_r4/Logical  logical_function=AND  inputs=2  en=off  latency=0  precision=Full  arith_type=Unsigned  n_bits=8  bin_pt=2  align_bp=on  dbl_ovrd=off  block_type=logical
      settings: xl_area=[1 0 0 1 0 0 0]  block_version=VER_STRING_GOES_HERE
  out0 : Outport  OutDataType=sfix(16)  OutScaling=2^0
  wires:
  in0/1 -> Logical3/1
  in1/1 -> Logical3/2
  Logical3/1 -> out0/1

== system ofdm_txrx_supermimo_coded/OFDM Tx MIMO/Training_Data/FlexibleMod/posedge2/Delay3 ==
  in0 : Inport  OutDataType=sfix(16)  OutScaling=2^0
  Delay3 : xbsIndex_r4/Delay  en=off  latency=1  dbl_ovrd=off  reg_retiming=off  block_type=delay
      settings: infoedit=Hardware notes: A delay line is a chain, each link of which is an SRL16 followed by a flip-flop. If register retiming is enabled, the delay line is a chain of flip-flops.  xl_area=[1 1 0 0 0 0 0]  block_version=VER_STRING_GOES_HERE
  out0 : Outport  OutDataType=sfix(16)  OutScaling=2^0
  wires:
  in0/1 -> Delay3/1
  Delay3/1 -> out0/1

== system ofdm_txrx_supermimo_coded/OFDM Tx MIMO/Training_Data/FlexibleMod/posedge2/Inverter2 ==
  in0 : Inport  OutDataType=sfix(16)  OutScaling=2^0
  Inverter2 : xbsIndex_r4/Inverter  en=off  latency=0  dbl_ovrd=off  block_type=inv
      settings: xl_area=[0 0 0 0 0 0 0]  block_version=VER_STRING_GOES_HERE
  out0 : Outport  OutDataType=sfix(16)  OutScaling=2^0
  wires:
  in0/1 -> Inverter2/1
  Inverter2/1 -> out0/1

== system ofdm_txrx_supermimo_coded/OFDM Tx MIMO/Training_Data/FlexibleMod/posedge2/Logical3 ==
  in0 : Inport  OutDataType=sfix(16)  OutScaling=2^0
  in1 : Inport  Port=2  OutDataType=sfix(16)  OutScaling=2^0
  Logical3 : xbsIndex_r4/Logical  logical_function=AND  inputs=2  en=off  latency=0  precision=Full  arith_type=Unsigned  n_bits=8  bin_pt=2  align_bp=on  dbl_ovrd=off  block_type=logical
      settings: xl_area=[1 0 0 1 0 0 0]  block_version=VER_STRING_GOES_HERE
  out0 : Outport  OutDataType=sfix(16)  OutScaling=2^0
  wires:
  in0/1 -> Logical3/1
  in1/1 -> Logical3/2
  Logical3/1 -> out0/1

== system ofdm_txrx_supermimo_coded/OFDM Tx MIMO/Training_Data/Training_Pilots/Scrambled_Pilot_Inserter1/Pilot Scrambling Sequence Gen ==
  rst : Inport  OutDataType=sfix(16)  OutScaling=2^0
  en : Inport  Port=2  OutDataType=sfix(16)  OutScaling=2^0
  Assert : xbsIndex_r4/Assert  Orientation=left  assert_type=on  type_source=Explicitly  arith_type=Boolean  n_bits=16  bin_pt=14  assert_rate=on  rate_source=Explicitly  period=2  output_port=on  block_type=assert
      settings: infoedit=Asserts a user-defined sample rate and/or type on a signal.<P><P>Hardware notes: In hardware this block costs nothing.  block_version=VER_STRING_GOES_HERE
  Assert1 : xbsIndex_r4/Assert  Orientation=left  assert_type=on  type_source=Explicitly  arith_type=Boolean  n_bits=16  bin_pt=14  assert_rate=on  rate_source=Explicitly  period=2  output_port=on  block_type=assert
      settings: infoedit=Asserts a user-defined sample rate and/or type on a signal.<P><P>Hardware notes: In hardware this block costs nothing.  block_version=VER_STRING_GOES_HERE
  Convert : xbsIndex_r4/Convert  arith_type=Boolean  n_bits=1  bin_pt=0  quantization=Truncate  overflow=Wrap  latency=0  dbl_ovrd=off  pipeline=off  block_type=convert
      settings: xl_area=[0, 0, 0, 0, 0, 0, 0]  block_version=VER_STRING_GOES_HERE
  Logical : xbsIndex_r4/Logical  Orientation=left  logical_function=XOR  inputs=2  en=off  latency=0  precision=Full  arith_type=Unsigned  n_bits=8  bin_pt=2  align_bp=on  dbl_ovrd=off  block_type=logical
      settings: xl_area=[0, 0, 0, 0, 0, 0, 0]  block_version=VER_STRING_GOES_HERE
  Logical1 : xbsIndex_r4/Logical  logical_function=AND  inputs=2  en=off  latency=0  precision=Full  arith_type=Unsigned  n_bits=8  bin_pt=2  align_bp=on  dbl_ovrd=off  block_type=logical
      settings: xl_area=[0, 0, 0, 0, 0, 0, 0]  block_version=VER_STRING_GOES_HERE
  Register{,1..6} (7 alike) : xbsIndex_r4/Register  init=1  rst=on  en=on  dbl_ovrd=off  block_type=register
      settings: xl_area=[0, 0, 0, 0, 0, 0, 0]  block_version=VER_STRING_GOES_HERE
  Q : Outport  OutDataType=sfix(16)  OutScaling=2^0
  wires:
  rst/1 -> Register/2, Register1/2, Register2/2, Register3/2, Register4/2, Register5/2, Register6/2
  Register6/1 -> Assert/1
  Logical1/1 -> Register/3, Register1/3, Register2/3, Register3/3, Register4/3, Register5/3, Register6/3
  Logical/1 -> Register/1, Convert/1
  Register5/1 -> Register6/1
  Register4/1 -> Register5/1
  Register3/1 -> Register4/1, Assert1/1
  Register2/1 -> Register3/1
  Register1/1 -> Register2/1
  Register/1 -> Register1/1
  Convert/1 -> Q/1
  Assert/1 -> Logical/1
  Assert1/1 -> Logical/2
  en/1 -> Logical1/1
  [TxReg_PilotScrambling] -> Logical1/2

== system ofdm_txrx_supermimo_coded/OFDM Tx MIMO/Training_Data/Training_Pilots/Scrambled_Pilot_Inserter1/Pilot Values ==
  16LSB : xbsIndex_r4/Slice  nbits=16  boolean_output=off  mode=Lower Bit Location + Width  bit1=0  base1=MSB of Input  bit0=0  base0=LSB of Input  dbl_ovrd=off  block_type=slice
      settings: infoedit="Extracts a given range of bits from each input sample and presents it at the output.  The output type is ordinarily unsigned with binary point at zero, but can be Boolean when the slice is one bit wide.<P><P>Hardware notes: In hardware this block costs nothing."  block_version=VER_STRING_GOES_HERE
  16MSB : xbsIndex_r4/Slice  nbits=16  boolean_output=off  mode=Upper Bit Location + Width  bit1=0  base1=MSB of Input  bit0=0  base0=LSB of Input  dbl_ovrd=off  block_type=slice
      settings: infoedit="Extracts a given range of bits from each input sample and presents it at the output.  The output type is ordinarily unsigned with binary point at zero, but can be Boolean when the slice is one bit wide.<P><P>Hardware notes: In hardware this block costs nothing."  block_version=VER_STRING_GOES_HERE
  Down Sample1 : xbsIndex_r4/Down Sample  sample_ratio=2  sample_phase="Last Value of Frame  (most efficient)"  en=off  latency=1  dbl_ovrd=off  block_type=dsamp
      settings: block_version=VER_STRING_GOES_HERE
  Down Sample3 : xbsIndex_r4/Down Sample  sample_ratio=2  sample_phase="Last Value of Frame  (most efficient)"  en=off  latency=1  dbl_ovrd=off  block_type=dsamp
      settings: block_version=VER_STRING_GOES_HERE
  Reinterpret : xbsIndex_r4/Reinterpret  force_arith_type=on  arith_type="Signed  (2's comp)"  force_bin_pt=on  bin_pt=15  block_type=cast
      settings: infoedit="Changes type of samples without altering their binary representation.<P><P>Hardware notes: In hardware this block costs nothing.<P><P>Example:  Suppose the input is 6 bits wide, signed, with 2 fractional bits, and the output is forced to unsigned with 0 fractional bits.  Then an input of -2.0 (1110.00 in binary 2's complement) becomes an output of 56 (111000 in binary)."  block_version=VER_STRING_GOES_HERE
  Reinterpret2 : xbsIndex_r4/Reinterpret  force_arith_type=on  arith_type="Signed  (2's comp)"  force_bin_pt=on  bin_pt=15  block_type=cast
      settings: infoedit="Changes type of samples without altering their binary representation.<P><P>Hardware notes: In hardware this block costs nothing.<P><P>Example:  Suppose the input is 6 bits wide, signed, with 2 fractional bits, and the output is forced to unsigned with 0 fractional bits.  Then an input of -2.0 (1110.00 in binary 2's complement) becomes an output of 56 (111000 in binary)."  block_version=VER_STRING_GOES_HERE
  val1 : Outport
  val2 : Outport  Port=2
  wires:
  [regTx_PilotToneValues] -> 16LSB/1, 16MSB/1
  16LSB/1 -> Reinterpret/1
  16MSB/1 -> Reinterpret2/1
  Reinterpret/1 -> Down Sample3/1
  Reinterpret2/1 -> Down Sample1/1
  Down Sample3/1 -> val2/1
  Down Sample1/1 -> val1/1

== system ofdm_txrx_supermimo_coded/OFDM Rx MIMO/AF Buffers/AF Buffers/Read Control/AF Preamble Counter/PreambleEn ==
  R : Inport  OutDataType=sfix(16)  OutScaling=2^0
  S : Inport  Port=2  OutDataType=sfix(16)  OutScaling=2^0
  Constant4 : xbsIndex_r4/Constant  arith_type=Boolean  const=1  n_bits=1  bin_pt=0  explicit_period=off  period=2  equ=P=C  opselect=C  inp2=PCIN>>17  opr=+  inp1=P  carry=CIN  dbl_ovrd=off  block_type=constant
      settings: block_version=VER_STRING_GOES_HERE
  Convert : xbsIndex_r4/Convert  arith_type=Boolean  n_bits=16  bin_pt=14  quantization=Truncate  overflow=Wrap  latency=0  dbl_ovrd=off  pipeline=off  block_type=convert
      settings: block_version=10.1.3
  Convert1 : xbsIndex_r4/Convert  arith_type=Boolean  n_bits=16  bin_pt=14  quantization=Truncate  overflow=Wrap  latency=0  dbl_ovrd=off  pipeline=off  block_type=convert
      settings: block_version=10.1.3
  Register1 : xbsIndex_r4/Register  init=0  rst=on  en=on  dbl_ovrd=off  block_type=register
      settings: xl_area=[5 10 0 0 0 0 0]  block_version=VER_STRING_GOES_HERE
  Q : Outport  OutDataType=sfix(16)  OutScaling=2^0
  wires:
  Convert1/1 -> Register1/3
  Convert/1 -> Register1/2
  S/1 -> Convert1/1
  Register1/1 -> Q/1
  R/1 -> Convert/1
  Constant4/1 -> Register1/1
